simulink model "TosAeb"  (R2021a)
settings: Solver=FixedStepDiscrete  StartTime=0.0  StopTime=inf  FixedStep=0.01  RelTol=1e-3
undefined symbols (not defined in the file or in its folder): ACCModSts, AccSts1, AEB_ObjectMovingOrientation, AEB_ObjectMovingState, CN_BTCActive, CN_CurrentGearR, CN_CurrentGearR_DHT, CN_PTCActive, CN_VDCActive, CN_VSC_EspOff, CN_VSC_FCW_AEB_Error, CN_VSC_FCW_AEB_NoDispay, CN_VSC_FCW_AEB_PerfmDgdtn, CN_VSC_FCW_AEB_TempUnAvlbl, CN_VSC_VehStandstill, CNaccelAvoidMax, CNaccelAvoidMin, CNbrakePedalPress, CNcomfortStrLeftAccelMax, CNcomfortStrLeftAccelMin, CNcomfortStrRightAccelMax, CNcomfortStrRightAccelMin, CNDrvDoorOpen, CNDrvModeEco, CNDrvModeNorm, CNDrvModeOther, CNDrvModeSport, CNDrvSBRreminder, CNNull, CNobjInvalidID, CNPassDoorOpen, CNPassSBRreminder, CNPEBSAccelNegInf, CNPEBSAccelNull, CNPEBSTimeInf, CNPEBSTimeNull, CNSTOPMAX, CNSTOPNULL, CNsubjWidth, CNSwtOFF, CNSwtON, CNTTCMAX, CNTTCNULL, CNVehConfigAEBCycNone, CNVehConfigAEBNone, CNVehConfigAEBPedNone, CNVehConfigFCWNone, CNVehConfigJANone, CollisionSeverity, FALSE, FCWSnvty, JACycObjCalcInfoDefaultParams, JAPedObjCalcInfoDefaultParams, JAVehObjCalcInfoDefaultParams, latCycObjCalcInfoDefaultParams, latPedObjCalcInfoDefaultParams, longObjCalcInfoDefaultParams, ModSts, movingStatus, ObjectType ... (+1112)
libraries: simulink x3031, AEBSFunctionLib x50, Ta_Library x33, AEBSStateMachineLib x23, Mylibrary x12, simulink_extras x7, ObjSelect_lib x3, dspstat3 x2
(part 75 of 102)

== system TosAeb/AEB_Objsel/TA_Processor/AEB_ObjSelect/TargetInfoCheck/Data_precess/TA_EffectCalculate/Obj_FilterFlgCalculate/Long_ObjFilter/TTR_Calculate/CalculateTTRAndTTM_else/LongitudinalTTRComputer/DetermineLongitudinalClosestSide ==
  note: This is to avoid
      | floating-point equality.
  note: VLgtRel = VLgtObj - VLgtHost
  note: If host drives faster than the object, or if VRel == 0 and the object is located in front of host,
      | then host's front will meet the object first.
  PosnLgt : Inport
  VLgtRel : Inport  Port=2
  Compare To Zero1 : Subsystem  mask(Compare To Zero){relop=<, OutDataTypeStr=boolean, ZeroCross=on}
      mask prompts: relop "Simulink:masks:Operator_MP"; OutDataTypeStr "Simulink:masks:OutputDataType_MP"; ZeroCross "Simulink:masks:EnableZerocrossingDetection_MP"
      mask help: Simulink:masks:CompareToZero_MD
      mask init: same as TosAeb/AEB_Objsel/TA_Processor/AEB_ObjSelect/TargetInfoCheck/Data_precess/TA_EffectCalculate/Obj_FilterFlgCalculate/Long_ObjFilter/TTR_Calculate/CalculateTTRAndTTM_else/ModifyTTRAtSameSpeedAndAcceleration/Compare To Zero
      mask TypeOptions relop: == | ~= | < | <= | >= | >
      mask TypeOptions OutDataTypeStr: Simulink:masks:uint8 | Simulink:masks:boolean
      mask Callback ZeroCross: sl('zeroCrossEnable', gcbh);
      mask Display: disp([relop,' 0'])
  Compare To Zero5 : Subsystem  mask(Compare To Zero){relop=>, OutDataTypeStr=boolean, ZeroCross=on}
      mask prompts: relop "Simulink:masks:Operator_MP"; OutDataTypeStr "Simulink:masks:OutputDataType_MP"; ZeroCross "Simulink:masks:EnableZerocrossingDetection_MP"
      mask help: Simulink:masks:CompareToZero_MD
      mask init: same as TosAeb/AEB_Objsel/TA_Processor/AEB_ObjSelect/TargetInfoCheck/Data_precess/TA_EffectCalculate/Obj_FilterFlgCalculate/Long_ObjFilter/TTR_Calculate/CalculateTTRAndTTM_else/ModifyTTRAtSameSpeedAndAcceleration/Compare To Zero
      mask TypeOptions relop: == | ~= | < | <= | >= | >
      mask TypeOptions OutDataTypeStr: Simulink:masks:uint8 | Simulink:masks:boolean
      mask Callback ZeroCross: sl('zeroCrossEnable', gcbh);
      mask Display: disp([relop,' 0'])
  Logop_1 : Logic  AllPortsSameDT=off  OutDataTypeStr=boolean
  Logop_2 : Logic  Operator=OR  AllPortsSameDT=off  OutDataTypeStr=boolean
  Logop_3 : Logic  Operator=NOT  AllPortsSameDT=off  OutDataTypeStr=boolean
  SizeChecker : Subsystem  mask(Size checker){UseInput=off, Limit=1e-2, DataTypeIn=single, Operator=>}
      mask prompts: UseInput "Take the limit as an input"; DataTypeIn "Data type:"; Operator "Relational operator:"
      mask help: The block tests the absolute value of the supplied signal against a specified value using the selected operator. If the signal conforms to the test, true is returned; else, false is returned. The limit can either be set in the mask or as an external signal.
      mask init: same as TosAeb/AEB_Objsel/TA_Processor/AEB_ObjSelect/TargetInfoCheck/Data_precess/TA_EffectCalculate/Obj_FilterFlgCalculate/Long_ObjFilter/TTR_Calculate/CalculateTTRAndTTM_else/ModifyTTRAtSameSpeedAndAcceleration/SizeChecker
      settings: AttributesFormatString=DT=%<DataTypeIn>
      mask Help: The block tests the absolute value of the supplied signal against a specified value using the selected operator. If the signal conforms to the test, true is returned; else, false is returned. The limit can either be set in the mask or as an external signal.
      mask Callback UseInput:
      | % Author: Jakob Andersson (jande573)
      | 
      | % Enable and disable the box for the limit variable depending on the status of the checkbox
      | if strcmp(get_param(gcb,'UseInput'),'on')
      |     % Disable the box
      |     set_param(gcb,'MaskEnables',{'on','off','on','on'}); % One setting for each parameter in this mask; needs to be updated if the parameters are changed
      | else
      |     % Enable the box
      |     set_param(gcb,'MaskEnables',{'on','on','on','on'}); % One setting for each parameter in this mask; needs to be updated if the parameters are changed
      | end
      mask Callback Limit:
      | % Author: Jakob Andersson (jande573)
      | 
      | % Define the default value to apply if the entered value is disallowed
      | default='1e-6';
      | 
      | % Evaluate the entered value
      | val=eval(get_param(gcb,'Limit'));
      | 
      | % Test the entered value and revert to the default if necessary
      | if isnumeric(val)==false
      |     % Set the default if the entered value did not evaluate to a numeric
      |     set_param(gcb,'Limit',default);
      |     errordlg('The limit must be numeric. The default value will be used instead.');
      | elseif val<0
      |     % Set the default if the entered value is negative
      |     set_param(gcb,'Limit',default);
      |     errordlg('The limit must be positive since it is compared to an absolute value. The default value will be used instead.');
      | end
      mask TypeOptions Operator:
      | Relational
      | Operator/Operator
      mask Callback Operator:
      | % Author: Jakob Andersson (jande573)
      | 
      | % Define the default value to apply if the entered value is disallowed
      | EnabledOperators={'==','~=','<','<=','>=','>'};
      | default='>';
      | 
      | % Evaluate the selected operator and determine if it is ok
      | ok=any(strcmp(get_param(gcb,'Operator'),EnabledOperators));
      | 
      | % Revert the operator if it is disabled
      | if ~ok
      |     % Set the default if the entered value did not evaluate to a numeric
      |     set_param(gcb,'Operator',default);
      |     errordlg('The selected operator is disabled. The default operator will be used instead.');
      | end
      mask Display:
      | % Display the mask for this block
      | % Author: Jakob Andersson (jande573)
      | 
      | % Transform the operator to TeX
      | switch Operator
      |     case '=='
      |         op='=';
      |     case '~='
      |         op='\neq';
      |     case '<='
      |         op='\leq';
      |     case '>='
      |         op='\geq';
      |     otherwise
      |         op=Operator;
      | end
      | 
      | % Display the correct mask depending on the number of inputs
      | if strcmp(UseInput,'on')
      |     % Display the block logic and the set limit
      |     disp(horzcat('|u| ',op,' a'),'texmode','on');
      | 
      |     % Display the port title
      |     port_label('input',1,'u');
      |     port_label('input',2,'a');
      | else
      |     % Display the block logic and the set limit
      |     disp(horzcat('|u| ',op,' ',num2str(Limit)),'texmode','on');
      | 
      |     % Display the port title
      |     port_label('input',1,'u');
      | end
  HostFrontIsClosest : Outport
  wires:
  Compare To Zero1/1(y) -> Logop_2/1  "HostIsFastest"
  Logop_1/1 -> Logop_2/2
  Compare To Zero5/1(y) -> Logop_1/2  "ObjectInFrontOfHost"
  PosnLgt/1 -> Compare To Zero5/1(u)
  Logop_3/1 -> Logop_1/1  "SameSpeed"
  VLgtRel/1 -> Compare To Zero1/1(u), SizeChecker/1(Signal)
  SizeChecker/1(TestOk) -> Logop_3/1
  Logop_2/1 -> HostFrontIsClosest/1  "HostFrontIsClosest"

== system TosAeb/AEB_Objsel/TA_Processor/AEB_ObjSelect/TargetInfoCheck/Data_precess/TA_EffectCalculate/Obj_FilterFlgCalculate/Long_ObjFilter/TTR_Calculate/CalculateTTRAndTTM_else/LongitudinalTTRComputer/FindWhichMotionDataToUse ==
  note: If host's front is closest to the object now, then we expect that it will still be closest when the edges of host and object meet.
      | Or, if the edges have already met in the past, we assume that the front was meeting the object first.
      | Consequently, MotionDataFrontClosest and EdgeDistanceFrontClosest is the normal setup for the front closest case.
      |
      | If the relative velocity changes direction before host and object meet, no solution for TTI will be found with the normal setup.
      | Then the alternative setup must be used. For the front closest case, MotionDataRearClosest and EdgeDistanceRearClosest
      | is the alternative setup.
  HostFrontIsClosest : Inport
  MotionDataFrontClosest : Inport  Port=2
  MotionDataRearClosest : Inport  Port=3
  EdgeDistanceFrontClosest : Inport  Port=4
  EdgeDistanceRearClosest : Inport  Port=5
  Switch_{1..4} (4 alike) : Switch  Criteria=u2 ~= 0
  MotionDataNormalSetup : Outport
  MotionDataAlternativeSetup : Outport  Port=2
  EdgeDistanceNormalSetup : Outport  Port=3
  EdgeDistanceAlternativeSetup : Outport  Port=4
  wires:
  EdgeDistanceRearClosest/1 -> Switch_4/1, Switch_2/3
  EdgeDistanceFrontClosest/1 -> Switch_2/1, [EdgeFront]
  Switch_4/1 -> EdgeDistanceAlternativeSetup/1  "EdgeDistanceAlternativeSetup"
  [EdgeFront] -> Switch_4/3
  [MotionFront] -> Switch_3/3
  MotionDataRearClosest/1 -> Switch_3/1, Switch_1/3
  Switch_3/1 -> MotionDataAlternativeSetup/1  "MotionDataAlternativeSetup"
  [FrontClosest] -> Switch_4/2
  [FrontClosest] -> Switch_3/2
  Switch_2/1 -> EdgeDistanceNormalSetup/1  "EdgeDistanceNormalSetup"
  [FrontClosest] -> Switch_2/2
  Switch_1/1 -> MotionDataNormalSetup/1  "MotionDataNormalSetup"
  HostFrontIsClosest/1 -> Switch_1/2, [FrontClosest]
  MotionDataFrontClosest/1 -> Switch_1/1, [MotionFront]

== system TosAeb/AEB_Objsel/TA_Processor/AEB_ObjSelect/TargetInfoCheck/Data_precess/TA_EffectCalculate/Obj_FilterFlgCalculate/Long_ObjFilter/TTR_Calculate/CalculateTTRAndTTM_else/LongitudinalTTRComputer/SelectTTI ==
  MovingTTI : Inport
  StationaryHostTTI : Inport  Port=2
  StationaryObjectTTI : Inport  Port=3
  MovingSolutionExists : Inport  Port=4
  StationaryHostSolutionExists : Inport  Port=5
  StationaryObjectSolutionExists : Inport  Port=6
  StoppingData : Inport  Port=7
  BothHostAndObjectWillStop : Subsystem  TreatAsAtomicUnit=on
  Bus Selector : BusSelector  OutputSignals=HostStopTime,ObjectStopTime
  CheckIfVehicleStops : Subsystem
  CheckIfVehicleStops1 : Subsystem
  HostAndObjectAreMoving : Subsystem  TreatAsAtomicUnit=on
  HostOnlyWillStop : Subsystem  TreatAsAtomicUnit=on
  If : If  NumInputs=3  IfExpression=u1~=0  ElseIfExpressions=u2~=0,u3~=0
  Logop_3 : Logic  AllPortsSameDT=off  OutDataTypeStr=boolean
  Merge : Merge  Inputs=4
  Merge1 : Merge  Inputs=4
  ObjectOnlyWillStop : Subsystem  TreatAsAtomicUnit=on
  TimeToIntersection : Outport
  SolutionExists : Outport  Port=2
  wires:
  [StationaryHostSolutionExists_in] -> BothHostAndObjectWillStop/6(StationaryHostSolutionExists)
  [StationaryObjectSolutionExists_in] -> BothHostAndObjectWillStop/5(StationaryObjectSolutionExists)
  If/4 -> [HostAndObjectAreMoving]
  If/3 -> [ObjectOnlyWillStop]
  [ObjectStops] -> If/3
  If/2 -> [HostOnlyWillStop]
  If/1 -> [HostAndObjectWillStop]
  StationaryHostTTI/1 -> [StatHostTTI], CheckIfVehicleStops1/1(StationaryTTI)
  StationaryObjectTTI/1 -> CheckIfVehicleStops/1(StationaryTTI), [StatObjTTI]
  Logop_3/1 -> If/1  "BothHostAndObjectWillStop"
  BothHostAndObjectWillStop/1(TimeToIntersection) -> Merge/1
  Merge/1 -> TimeToIntersection/1  "TimeToIntersection"
  [ObjStopTime] -> BothHostAndObjectWillStop/3(ObjectStopTime)
  [StatObjTTI] -> ObjectOnlyWillStop/1(StationaryObjectTTI)
  [StatHostTTI] -> HostOnlyWillStop/1(StationaryHostTTI)
  [HostAndObjectWillStop] -> BothHostAndObjectWillStop/Action
  [StatHostTTI] -> BothHostAndObjectWillStop/2(StationaryHostTTI)
  [StatObjTTI] -> BothHostAndObjectWillStop/1(StationaryObjectTTI)
  [HostAndObjectAreMoving] -> HostAndObjectAreMoving/Action
  [HostOnlyWillStop] -> HostOnlyWillStop/Action
  [ObjectOnlyWillStop] -> ObjectOnlyWillStop/Action
  MovingTTI/1 -> [MovingTTI], HostAndObjectAreMoving/1(MovingTTI)
  Bus Selector/1 -> BothHostAndObjectWillStop/4(HostStopTime), CheckIfVehicleStops1/3(StopTime)  "<HostStopTime>"
  CheckIfVehicleStops1/1(VehicleStops) -> Logop_3/2, If/2  "HostStops"
  CheckIfVehicleStops/1(VehicleStops) -> [ObjectStops], Logop_3/1  "ObjectStops"
  StoppingData/1 -> Bus Selector/1
  Bus Selector/2 -> [ObjStopTime]  "<ObjectStopTime>"
  [ObjStopTime] -> CheckIfVehicleStops/3(StopTime)
  [MovingTTI] -> CheckIfVehicleStops/2(MovingTTI)
  [MovingTTI] -> CheckIfVehicleStops1/2(MovingTTI)
  MovingSolutionExists/1 -> [MovingSolutionExists_in]
  StationaryHostSolutionExists/1 -> [StationaryHostSolutionExists_in]
  StationaryObjectSolutionExists/1 -> [StationaryObjectSolutionExists_in]
  [StationaryObjectSolutionExists_in] -> ObjectOnlyWillStop/2(StationaryObjectSolutionExists)
  [StationaryHostSolutionExists_in] -> HostOnlyWillStop/2(StationaryHostSolutionExists)
  [MovingSolutionExists_in] -> HostAndObjectAreMoving/2(MovingSolutionExists)
  BothHostAndObjectWillStop/2(SolutionExists) -> [HostAndObjectSolution]
  HostOnlyWillStop/2(SolutionExists) -> [HostSolution]
  ObjectOnlyWillStop/2(SolutionExists) -> [ObjectSolution]
  HostAndObjectAreMoving/2(SolutionExists) -> Merge1/4
  HostOnlyWillStop/1(TimeToIntersection) -> Merge/2
  ObjectOnlyWillStop/1(TimeToIntersection) -> Merge/3
  HostAndObjectAreMoving/1(TimeToIntersection) -> Merge/4
  Merge1/1 -> SolutionExists/1  "SolutionExists"
  [HostAndObjectSolution] -> Merge1/1
  [HostSolution] -> Merge1/2
  [ObjectSolution] -> Merge1/3

== system TosAeb/AEB_Objsel/TA_Processor/AEB_ObjSelect/TargetInfoCheck/Data_precess/TA_EffectCalculate/Obj_FilterFlgCalculate/Long_ObjFilter/TTR_Calculate/CalculateTTRAndTTM_else/ModifyTTRAtSameSpeedAndAcceleration/Compare To Zero ==
  u : Inport
  Compare : RelationalOperator  Operator=<  OutDataTypeStr=boolean  RndMeth=Nearest  InputSameDT=on
  Constant : Constant  DisableCoverage=on  Value=0  OutDataTypeStr=Inherit: Inherit via back propagation  PreserveConstantTs=on
  y : Outport
  wires:
  Constant/1 -> Compare/2
  u/1 -> Compare/1
  Compare/1 -> y/1

== system TosAeb/AEB_Objsel/TA_Processor/AEB_ObjSelect/TargetInfoCheck/Data_precess/TA_EffectCalculate/Obj_FilterFlgCalculate/Long_ObjFilter/TTR_Calculate/CalculateTTRAndTTM_else/ModifyTTRAtSameSpeedAndAcceleration/Compare To Zero1 ==
  u : Inport
  Compare : RelationalOperator  Operator=<  OutDataTypeStr=boolean  RndMeth=Nearest  InputSameDT=on
  Constant : Constant  DisableCoverage=on  Value=0  OutDataTypeStr=Inherit: Inherit via back propagation  PreserveConstantTs=on
  y : Outport
  wires:
  Constant/1 -> Compare/2
  u/1 -> Compare/1
  Compare/1 -> y/1

== system TosAeb/AEB_Objsel/TA_Processor/AEB_ObjSelect/TargetInfoCheck/Data_precess/TA_EffectCalculate/Obj_FilterFlgCalculate/Long_ObjFilter/TTR_Calculate/CalculateTTRAndTTM_else/ModifyTTRAtSameSpeedAndAcceleration/SizeChecker ==
  Signal : Inport  OutDataTypeStr=DataTypeIn
      settings: AttributesFormatString=DT=%<OutDataTypeStr>
  Abs : Abs  SaturateOnIntegerOverflow=on
  LimitSource : Constant  Value=Limit  OutDataTypeStr=Inherit: Inherit via back propagation
      settings: AttributesFormatString=DT=%<OutDataTypeStr>
  Relational Operator : RelationalOperator  Operator=<  OutDataTypeStr=boolean  RndMeth=Nearest  InputSameDT=on
      settings: AttributesFormatString=InputSameDT=%<InputSameDT>
  TestOk : Outport  OutDataTypeStr=boolean
      settings: AttributesFormatString=DT=%<OutDataTypeStr>
  wires:
  Relational Operator/1 -> TestOk/1  "TestOk"
  Signal/1 -> Abs/1  "Signal"
  LimitSource/1 -> Relational Operator/2  "LimitSource"
  Abs/1 -> Relational Operator/1  "AbsoluteSignal"

== system TosAeb/AEB_Objsel/TA_Processor/AEB_ObjSelect/TargetInfoCheck/Data_precess/TA_EffectCalculate/Obj_FilterFlgCalculate/Long_ObjFilter/TTR_Calculate/CalculateTTRAndTTM_else/ModifyTTRAtSameSpeedAndAcceleration/SizeChecker1 ==
  Signal : Inport  OutDataTypeStr=DataTypeIn
      settings: AttributesFormatString=DT=%<OutDataTypeStr>
  Abs : Abs  SaturateOnIntegerOverflow=on
  LimitSource : Constant  Value=Limit  OutDataTypeStr=Inherit: Inherit via back propagation
      settings: AttributesFormatString=DT=%<OutDataTypeStr>
  Relational Operator : RelationalOperator  Operator=<  OutDataTypeStr=boolean  RndMeth=Nearest  InputSameDT=on
      settings: AttributesFormatString=InputSameDT=%<InputSameDT>
  TestOk : Outport  OutDataTypeStr=boolean
      settings: AttributesFormatString=DT=%<OutDataTypeStr>
  wires:
  Relational Operator/1 -> TestOk/1  "TestOk"
  Signal/1 -> Abs/1  "Signal"
  LimitSource/1 -> Relational Operator/2  "LimitSource"
  Abs/1 -> Relational Operator/1  "AbsoluteSignal"

== system TosAeb/AEB_Objsel/TA_Processor/AEB_ObjSelect/TargetInfoCheck/Data_precess/TA_EffectCalculate/Obj_FilterFlgCalculate/Long_ObjFilter/TTR_Calculate/CalculateTTRAndTTM_else/Obj_AbsInfo/InforForTTICalculation ==
  note: The MotionDataFrontClosest bus is used when host's front will meet the object first,
      | i.e. when host has the highest speed.
      | This set of data is also used when VRel == 0 and the object is located in front of host.
  note: The MotionDataRearClosest bus is used when host's rear will meet the object first,
      | i.e. when the object has the highest speed.
      | This set of data is also used when VRel == 0 and the object is located behind host.
  VRel : Inport
  VLgtObj : Inport  Port=2
  VLgtHost : Inport  Port=3
  ARel : Inport  Port=4
  ALgtObj : Inport  Port=5
  ALgtHost : Inport  Port=6
  Bus Creator : BusCreator  Inputs=6
  Bus Creator1 : BusCreator  Inputs=6
  Unary Minus{,1..5} (6 alike) : UnaryMinus
  MotionDataFrontClosest : Outport
  MotionDataRearClosest : Outport  Port=2
  wires:
  [ALgtHost] -> Bus Creator1/6  "ARelStationaryObject"
  [VRel] -> Unary Minus2/1
  Bus Creator/1 -> MotionDataFrontClosest/1  "MotionDataFrontClosest"
  Unary Minus1/1 -> Bus Creator/6  "ARelStationaryObject"
  ALgtObj/1 -> Bus Creator/5, [ALgtObj]  "ARelStationaryHost"
  [ALgtObj] -> Unary Minus5/1
  VLgtObj/1 -> Bus Creator/2, [VLgtObj]  "VRelStationaryHost"
  Unary Minus3/1 -> Bus Creator1/2  "VRelStationaryHost"
  ALgtHost/1 -> [ALgtHost], Unary Minus1/1
  [VLgtObj] -> Unary Minus3/1
  Unary Minus5/1 -> Bus Creator1/5  "ARelStationaryHost"
  Unary Minus2/1 -> Bus Creator1/1  "VRel"
  Unary Minus4/1 -> Bus Creator1/4  "ARel"
  [VLgtHost] -> Bus Creator1/3  "VRelStationaryObject"
  ARel/1 -> Unary Minus4/1, Bus Creator/4  "ARel"
  Unary Minus/1 -> Bus Creator/3  "VRelStationaryObject"
  VRel/1 -> [VRel], Bus Creator/1  "VRel"
  Bus Creator1/1 -> MotionDataRearClosest/1  "MotionDataRearClosest"
  VLgtHost/1 -> [VLgtHost], Unary Minus/1

== system TosAeb/AEB_Objsel/TA_Processor/AEB_ObjSelect/TargetInfoCheck/Data_precess/TA_EffectCalculate/Obj_FilterFlgCalculate/Long_ObjFilter/TTR_Calculate/CalculateTTRAndTTM_else/Obj_AbsInfo/LongitudinalEdgeToEdgeDistances ==
  note: This subsystem calculates the edge to edge distances for the following two cases:
      | 	* Host's front will meet the object first. This happens when host has the highest speed.
      | 	* Host's rear end will meet the object first. This happens when the object has the highest speed.
  TA_ObjInfo : Inport
  Add2 : Sum
  Bus Selector : BusSelector  OutputSignals=TA_ObsDx
  Constant : Constant  Value=P_TA_PrmForVehLongth
  Rename : Subsystem  mask(Rename){}
      mask help: Block intended for fooling the model advisor, which want the signal names to match the port names and which only allows signal renaming in combination with transformative operations. The block has no effect on the signal and is intended to replace, e.g., gain blocks with one as its multiplication factor.
      mask Help: Block intended for fooling the model advisor, which want the signal names to match the port names and which only allows signal renaming in combination with transformative operations. The block has no effect on the signal and is intended to replace, e.g., gain blocks with one as its multiplication factor.
      mask Display:
      | % Author: Jakob Andersson (jande573)
      | 
      | % Display the block title
      | disp('Rename');
  Rename1 : Subsystem  mask(Rename){}
      mask help: Block intended for fooling the model advisor, which want the signal names to match the port names and which only allows signal renaming in combination with transformative operations. The block has no effect on the signal and is intended to replace, e.g., gain blocks with one as its multiplication factor.
      mask Help: Block intended for fooling the model advisor, which want the signal names to match the port names and which only allows signal renaming in combination with transformative operations. The block has no effect on the signal and is intended to replace, e.g., gain blocks with one as its multiplication factor.
      mask Display:
      | % Author: Jakob Andersson (jande573)
      | 
      | % Display the block title
      | disp('Rename');
  Unary Minus6 : UnaryMinus
  CloseEdgeDistanceFrontClosest : Outport
  CloseEdgeDistanceRearClosest : Outport  Port=2
  FarEdgeDistanceFrontClosest : Outport  Port=3
  wires:
  Rename1/1(Name_out) -> FarEdgeDistanceFrontClosest/1  "FarEdgeDistanceFrontClosest"
  Unary Minus6/1 -> CloseEdgeDistanceRearClosest/1  "CloseEdgeDistanceRearClosest"
  Rename/1(Name_out) -> CloseEdgeDistanceFrontClosest/1  "CloseEdgeDistanceFrontClosest"
  Add2/1 -> Rename1/1(Name_in), Unary Minus6/1  "DistanceFromHostRear"
  Constant/1 -> Add2/2
  TA_ObjInfo/1 -> Bus Selector/1
  Bus Selector/1 -> Add2/1, Rename/1(Name_in)  "<TA_ObsDx>"

== system TosAeb/AEB_Objsel/TA_Processor/AEB_ObjSelect/TargetInfoCheck/Data_precess/TA_EffectCalculate/Obj_FilterFlgCalculate/Long_ObjFilter/TTR_Calculate/CalculateTTRAndTTM_else/Obj_AbsInfo/LongitudinalMotionData ==
  TA_ObjInfo : Inport
  VehSelfData : Inport  Port=2
  Bus Selector : BusSelector  OutputSignals=VehSelf.ABS_VehicleSpeed,VehSelf.AX1
  Bus Selector2 : BusSelector  OutputSignals=TA_ObsVx,TA_ObsVxAbs,TA_ObsAx,TA_ObsAxAbs
  Subsystem : Subsystem  Commented=on
  Obj_RefVx : Outport
  Obj_AbsVx : Outport  Port=2
  Subj_Vx : Outport  Port=3
  Obj_RefAcclx : Outport  Port=4
  Obj_AbsAcclx : Outport  Port=5
  Subj_Ax : Outport  Port=6
  wires:
  TA_ObjInfo/1 -> Bus Selector2/1
  Bus Selector2/1 -> Obj_RefVx/1  "<TA_ObsVx>"
  Bus Selector2/2 -> Obj_AbsVx/1  "<TA_ObsVxAbs>"
  Bus Selector2/3 -> Obj_RefAcclx/1  "<TA_ObsAx>"
  Bus Selector2/4 -> Obj_AbsAcclx/1  "<TA_ObsAxAbs>"
  VehSelfData/1 -> Bus Selector/1
  Bus Selector/2 -> Subj_Ax/1  "<AX1>"
  Bus Selector/1 -> Subj_Vx/1  "<ABS_VehicleSpeed>"

== system TosAeb/AEB_Objsel/TA_Processor/AEB_ObjSelect/TargetInfoCheck/Data_precess/TA_EffectCalculate/Obj_FilterFlgCalculate/Long_ObjFilter/TTR_Calculate/CalculateTTRAndTTM_else/Obj_AbsInfo/StoppingDataForTTICalculation ==
  note: 计算自车刹停时间、刹停距离
      | 计算目标刹停时间、刹停距离
  VLgtObj : Inport
  ALgtObj : Inport  Port=2
  VLgtHost : Inport  Port=3
  ALgtHost : Inport  Port=4
  TA_ObjInfo : Inport  Port=5
  Bus Creator : BusCreator  Inputs=4
  Bus Selector : BusSelector  OutputSignals=TA_ObsState
  Compare To Constant : simulink/Logic and Bit Operations/Compare To Constant  relop===  const=2  OutDataTypeStr=boolean
  Compare To Constant1 : simulink/Logic and Bit Operations/Compare To Constant  relop===  const=3  OutDataTypeStr=boolean
  Constant1 : Constant  Value=single(0)
  Constant2 : Constant  Value=single(0.01)
  Constant3 : Constant  Value=single(0)
  Constant4 : Constant  Value=single(0)
  Gain_1 : Gain  Gain=-1  ParamDataTypeStr=single  OutDataTypeStr=single
  Gain_2 : Gain  Gain=-1  ParamDataTypeStr=single  OutDataTypeStr=single
  LimitedSafeDivide : Subsystem
  LimitedSafeDivide1 : Subsystem
  LinearObjectPositionPredictor : Subsystem
  LinearObjectPositionPredictor1 : Subsystem
  Logical Operator : Logic  Operator=OR  AllPortsSameDT=off  OutDataTypeStr=boolean
  Switch : Switch  Criteria=u2 ~= 0
  Switch1 : Switch  Criteria=u2 ~= 0
  StoppingData : Outport
  wires:
  Gain_2/1 -> LimitedSafeDivide1/2(Denominator)  "Deceleration"
  Gain_1/1 -> LimitedSafeDivide/2(Denominator)  "Deceleration"
  VLgtObj/1 -> LinearObjectPositionPredictor1/2(V), LimitedSafeDivide/1(Numerator)
  LimitedSafeDivide/1(LimitedQuotient) -> LinearObjectPositionPredictor1/4(Ti), Switch/3  "ObjectStopTime"
  ALgtObj/1 -> LinearObjectPositionPredictor1/3(A), Gain_1/1
  VLgtHost/1 -> LimitedSafeDivide1/1(Numerator), LinearObjectPositionPredictor/2(V)
  LimitedSafeDivide1/1(LimitedQuotient) -> [HostStopTime], Bus Creator/3  "HostStopTime"
  ALgtHost/1 -> Gain_2/1, LinearObjectPositionPredictor/3(A)
  LinearObjectPositionPredictor/1(PosnPred) -> Bus Creator/4  "HostDeltaPos"
  Constant4/1 -> LinearObjectPositionPredictor/1(Posn)
  LinearObjectPositionPredictor1/1(PosnPred) -> Switch1/3  "ObjectDeltaPos"
  Constant1/1 -> LinearObjectPositionPredictor1/1(Posn)
  [HostStopTime] -> LinearObjectPositionPredictor/4(Ti)
  Bus Creator/1 -> StoppingData/1  "StoppingData"
  [Stationary] -> Switch/2
  Constant2/1 -> Switch/1
  [Stationary] -> Switch1/2
  Constant3/1 -> Switch1/1
  Switch1/1 -> Bus Creator/1  "ObjectDeltaPos"
  Switch/1 -> Bus Creator/2  "ObjectStopTime"
  TA_ObjInfo/1 -> Bus Selector/1
  Compare To Constant/1 -> Logical Operator/1
  Logical Operator/1 -> [Stationary]
  Compare To Constant1/1 -> Logical Operator/2
  Bus Selector/1 -> Compare To Constant1/1, Compare To Constant/1  "<TA_ObsState>"

== system TosAeb/AEB_Objsel/TA_Processor/AEB_ObjSelect/TargetInfoCheck/Data_precess/TA_EffectCalculate/Obj_FilterFlgCalculate/Long_ObjFilter/TTR_Calculate/CalculateTTRAndTTM_else/SetCorrectSign/Compare To Zero1 ==
  u : Inport
  Compare : RelationalOperator  Operator=<  OutDataTypeStr=boolean  RndMeth=Nearest  InputSameDT=on
  Constant : Constant  DisableCoverage=on  Value=0  OutDataTypeStr=Inherit: Inherit via back propagation  PreserveConstantTs=on
  y : Outport
  wires:
  Constant/1 -> Compare/2
  u/1 -> Compare/1
  Compare/1 -> y/1

== system TosAeb/AEB_Objsel/TA_Processor/AEB_ObjSelect/TargetInfoCheck/Data_precess/TA_EffectCalculate/Obj_FilterFlgCalculate/Long_ObjFilter/TTR_Calculate/CalculateTTRAndTTM_else/SetCorrectSign/Compare To Zero2 ==
  u : Inport
  Compare : RelationalOperator  Operator=<  OutDataTypeStr=boolean  RndMeth=Nearest  InputSameDT=on
  Constant : Constant  DisableCoverage=on  Value=0  OutDataTypeStr=Inherit: Inherit via back propagation  PreserveConstantTs=on
  y : Outport
  wires:
  Constant/1 -> Compare/2
  u/1 -> Compare/1
  Compare/1 -> y/1

== system TosAeb/AEB_Objsel/TA_Processor/AEB_ObjSelect/TargetInfoCheck/Data_precess/TA_EffectCalculate/ValideateObj/ObjPos_Calculate/ObjPos_Calculateelse/InPath_Cal/DetermineInPath/Compare To Zero ==
  u : Inport
  Compare : RelationalOperator  Operator=<  OutDataTypeStr=boolean  RndMeth=Nearest  InputSameDT=on
  Constant : Constant  DisableCoverage=on  Value=0  OutDataTypeStr=Inherit: Inherit via back propagation  PreserveConstantTs=on
  y : Outport
  wires:
  Constant/1 -> Compare/2
  u/1 -> Compare/1
  Compare/1 -> y/1

== system TosAeb/AEB_Objsel/TA_Processor/AEB_ObjSelect/TargetInfoCheck/Data_precess/TA_EffectCalculate/ValideateObj/ObjPos_Calculate/ObjPos_Calculateelse/InPath_Cal/DetermineInPath/LimitedSafeDivide ==
  Numerator : Inport
  Denominator : Inport  Port=2
  Constant1 : Constant  Value=-1000  OutDataTypeStr=single
  Constant5 : Constant  Value=1000  OutDataTypeStr=single
  SafeDivide : Subsystem  TreatAsAtomicUnit=on  mask(Safe divide){DataType=single, DataTypeMax=0, DataTypeMin=0}
      mask prompts: DataType "Data type:"
      mask help:
      | This block offers division protected from division by zero.
      |
      | When division by zero is detected, a very large number will be returned with the same sign as the numerator.
      |
      | In the case zero divided by zero, the output has been defined as zero.
      |
      | Both floating-point numbers and integers are supported.
      mask init: same as TosAeb/AEB_Objsel/TA_Processor/PreProcessor/VehiclePreProcessor/CalculateCurvature/SafeDivide
      settings: RTWSystemCode=Inline
      mask Help:
      | This block offers division protected from division by zero.
      | 
      | When division by zero is detected, a very large number will be returned with the same sign as the numerator.
      | 
      | In the case zero divided by zero, the output has been defined as zero.
      | 
      | Both floating-point numbers and integers are supported.
      mask Display:
      | % Author: Jakob Andersson (jande573)
      | 
      | % Display the allowed data type
      | disp(DataType);
      | 
      | % Display the port symbols
      | port_label('input',1,'\bullet','texmode','on');
      | port_label('input',2,'\div','texmode','on');
  Saturation Dynamic : Subsystem  mask(Saturation Dynamic){OutMin=[], OutMax=[], OutDataTypeStr=Inherit: Same as second input, OutputDataTypeScalingMode=Same as second input, OutDataType=fixdt(1, 16), OutScaling=2^-10, LockScale=off, RndMeth=Floor, DoSatur=off}
      mask prompts: OutMin "Simulink:masks:OutputMinimum_MP"; OutMax "Simulink:masks:OutputMaximum_MP"; OutDataTypeStr "Simulink:masks:OutputDataType_MP"; OutputDataTypeScalingMode "Simulink:masks:OutputDataTypeMode_MP"; OutDataType "Simulink:masks:OutputDataTypeegFixdt116Fixdtsingle_MP"; OutScaling "Simulink:masks:OutputScalingSlopeEg29OrSlopeBias_MP"; LockScale "Simulink:masks:LockOutputDataTypeAgainstFxpTools_MP"; RndMeth "Simulink:masks:IntegerRoundingMode_MP"; DoSatur "Simulink:masks:SaturateOnIntegerOverflow_MP"
      mask help: Simulink:masks:SaturationDynamic_MD
      mask init:
      | blkH = gcbh;
      | blkName = getfullname(blkH);
      | 
      | switch_blks = {'Switch','Switch2'}; 
      | for i = 1:length(switch_blks)
      |   set_param([blkName,'/',switch_blks{i}], ...
      |       'RndMeth', RndMeth, ...
      | 	  'DoSatur', DoSatur);
      | end
      | 
      | propBlk = [blkName, '/Data Type Propagation'];
      | 
      | if strcmp(OutputDataTypeScalingMode, 'Same as second input')
      |     set_param(propBlk, 'PropDataTypeMode', 'Inherit via propagation rule', ...
      |                        'PropScalingMode',  'Inherit via propagation rule');
      | else
      |     scaling = OutScaling;
      |     scalingMode = 'Specify via dialog';
      |     switch OutputDataTypeScalingMode
      |       case 'double'
      |         dataType = 'fixdt(''double'')';
      |       case 'single'
      |         dataType = 'fixdt(''single'')';
      |       case 'int8'
      |         dataType = 'fixdt(1,8,0)';
      |       case 'uint8'
      |         dataType = 'fixdt(0,8,0)';
      |       case 'int16'
      |         dataType = 'fixdt(1,16,0)';
      |       case 'uint16'
      |         dataType = 'fixdt(0,16,0)';
      |       case 'int32'
      |         dataType = 'fixdt(1,32,0)';
      |       case 'uint32'
      |         dataType = 'fixdt(0,32,0)';
      |       case 'Specify via dialog'
      |         dataType = OutDataType;
      |         if ~isempty(OutDataTypeStr) && isa(OutDataTypeStr,'Simulink.NumericType') 
      |           if strcmp(OutDataTypeStr.DataTypeMode, 'Fixed-point: unspecified scaling') 
      |             scalingMode = 'Obtain via best precision';
      |             range = [OutMin OutMax];
      |             range = range(~isinf(range));
      |             set_param(propBlk, 'ValuesUsedBestPrec', mat2str(range));
      |           end
      |         end
      |     end
      |     
      |     set_param(propBlk, 'PropDataTypeMode', 'Specify via dialog', ...
      |                        'PropScalingMode',  scalingMode, ...
      |                        'PropDataType',     dataType, ...
      |                        'PropScaling',      scaling);
      | end
      mask Callback OutDataTypeStr: fixptdialog(gcbh)
      mask TypeOptions OutputDataTypeScalingMode: double | single | int8 | uint8 | int16 | uint16 | int32 | uint32 | Specify via dialog | Same as second input
      mask Callback OutputDataTypeScalingMode: fixptdialog(gcbh)
      mask Callback OutDataType: fixptdialog(gcbh)
      mask TypeOptions RndMeth: Simulink:masks:Ceiling | Simulink:masks:Convergent | Simulink:masks:Floor | Simulink:masks:Nearest | Simulink:masks:Round | Simulink:masks:Simplest | Simulink:masks:Zero
      mask Display: plot([0.15 0.85],[.5 .5],[.5 .5],[0.05 0.95],0.15+0.7*[0 .25 .75 1],0.05+0.9*[.25 .25 .75 .75]);
  LimitedQuotient : Outport
  wires:
  Constant1/1 -> Saturation Dynamic/3(lo)
  Constant5/1 -> Saturation Dynamic/1(up)
  Saturation Dynamic/1(y) -> LimitedQuotient/1
  SafeDivide/1(Quotient) -> Saturation Dynamic/2(u)
  Denominator/1 -> SafeDivide/2(Denominator)
  Numerator/1 -> SafeDivide/1(Numerator)

== system TosAeb/AEB_Objsel/TA_Processor/AEB_ObjSelect/TargetInfoCheck/Data_precess/TA_EffectCalculate/ValideateObj/ObjPos_Calculate/ObjPos_Calculateelse/InPath_Cal/DetermineInPath/PredictHostLateralPositionAtTTR ==
  PredictionTime : Inport  PortDimensions=1
  PahEstimn : Inport  Port=2
  CalculateHeading : Subsystem  Commented=on
  PositionAndDerivative : MATLAB Function  TreatAsAtomicUnit=on
  PositionAndDerivative1 : MATLAB Function  TreatAsAtomicUnit=on
  SegmentComponents : Subsystem
  SelectSegment : Subsystem
  PredHostPosnLgt : Outport
  PredHostPosnLat : Outport  Port=2
  PredHostHeading : Outport  Port=3
  wires:
  [tau] -> PositionAndDerivative/5(tau)
  PositionAndDerivative1/1(y) -> PredHostPosnLat/1  "PredHostPosnLat"
  PositionAndDerivative/1(x) -> PredHostPosnLgt/1  "PredHostPosnLgt"
  SegmentComponents/9(tau) -> [tau], PositionAndDerivative1/5(tau)
  SegmentComponents/8(P3y) -> PositionAndDerivative1/4(P3)
  SegmentComponents/7(P2y) -> PositionAndDerivative1/3(P2)
  SegmentComponents/6(P1y) -> PositionAndDerivative1/2(P1)
  SegmentComponents/5(P0y) -> PositionAndDerivative1/1(P0)
  SegmentComponents/4(P3x) -> PositionAndDerivative/4(P3)
  SegmentComponents/3(P2x) -> PositionAndDerivative/3(P2)
  SegmentComponents/2(P1x) -> PositionAndDerivative/2(P1)
  SegmentComponents/1(P0x) -> PositionAndDerivative/1(P0)
  PahEstimn/1 -> SelectSegment/1(PahEstimn), SegmentComponents/1(Segment), CalculateHeading/1(PathEstimn)  "PahEstimn"
  PredictionTime/1 -> SegmentComponents/2(PredictionTime), SelectSegment/2(Time)  "PredictionTime"
  PositionAndDerivative/2(dx) -> [dx]
  PositionAndDerivative1/2(dy) -> [dy]
  CalculateHeading/1(PredHostHeading) -> PredHostHeading/1
  [dx] -> CalculateHeading/3(dx)
  [dy] -> CalculateHeading/4(dy)
  SelectSegment/1(SegmentIndex) -> SegmentComponents/3(SegmentIndex)

== system TosAeb/AEB_Objsel/TA_Processor/AEB_ObjSelect/TargetInfoCheck/Data_precess/TA_EffectCalculate/ValideateObj/ObjPos_Calculate/ObjPos_Calculateelse/InPath_Cal/DetermineInPath/PredictObjectLateralPositionAtTTR ==
  note: Constant acceleration prediction:
      | pos_pred = pos + vel*time + acc*time^2/2
      | vel_pred = vel + acc*time
      | acc_pred = acc
  Posn : Inport  OutDataTypeStr=single
      settings: AttributesFormatString=DT=%<OutDataTypeStr>
  V : Inport  Port=2  OutDataTypeStr=single
      settings: AttributesFormatString=DT=%<OutDataTypeStr>
  A : Inport  Port=3  OutDataTypeStr=single
      settings: AttributesFormatString=DT=%<OutDataTypeStr>
  Ti : Inport  Port=4  OutDataTypeStr=single
      settings: AttributesFormatString=DT=%<OutDataTypeStr>
  Constant : Constant  Value=single(0.5)  OutDataTypeStr=single
      settings: AttributesFormatString=DT=%<OutDataTypeStr>
  Product1 : Product  RndMeth=Zero
  Product4 : Product  Inputs=**  RndMeth=Zero
  Product5 : Product  Inputs=***  RndMeth=Zero
  Product6 : Product  Inputs=**  RndMeth=Zero
  Rename : Subsystem  mask(Rename){}
      mask help: Block intended for fooling the model advisor, which want the signal names to match the port names and which only allows signal renaming in combination with transformative operations. The block has no effect on the signal and is intended to replace, e.g., gain blocks with one as its multiplication factor.
      mask Help: Block intended for fooling the model advisor, which want the signal names to match the port names and which only allows signal renaming in combination with transformative operations. The block has no effect on the signal and is intended to replace, e.g., gain blocks with one as its multiplication factor.
      mask Display:
      | % Author: Jakob Andersson (jande573)
      | 
      | % Display the block title
      | disp('Rename');
  Sum3 : Sum
  Sum6 : Sum  Inputs=+++
  PosnPred : Outport  OutDataTypeStr=single
      settings: AttributesFormatString=DT=%<OutDataTypeStr>
  VPred : Outport  Port=2  OutDataTypeStr=single
      settings: AttributesFormatString=DT=%<OutDataTypeStr>
  APred : Outport  Port=3  OutDataTypeStr=single
      settings: AttributesFormatString=DT=%<OutDataTypeStr>
  wires:
  Rename/1(Name_out) -> APred/1  "APred"
  Product5/1 -> Sum6/3  "PositionChangeFromAcceleration"
  Constant/1 -> Product5/3  "Denominator"
  Posn/1 -> Sum6/1  "Posn"
  V/1 -> Product4/1, Sum3/1  "V"
  Ti/1 -> Product1/2, Product1/1, Product4/2, Product6/1  "Ti"
  A/1 -> Product6/2, Rename/1(Name_in), Product5/1  "A"
  Product4/1 -> Sum6/2  "PositionChangeFromVelocity"
  Product1/1 -> Product5/2  "TimeSquared"
  Sum6/1 -> PosnPred/1  "PosnPred"
  Product6/1 -> Sum3/2  "VelocityChangeFromAcceleration"
  Sum3/1 -> VPred/1  "VPred"

== system TosAeb/AEB_Objsel/TA_Processor/AEB_ObjSelect/TargetInfoCheck/Data_precess/TA_EffectCalculate/ValideateObj/ValidObj_PreProcess/If Action Subsystem1/CheckPosition/Subsystem/dxCalc_if ==
  ObsDx : Inport
  Action Port : ActionPort  ActionPortLabel=if(u1 > 0)
  Obsdx : Outport
  wires:
  ObsDx/1 -> Obsdx/1  "ObjPreSelectCond"

== system TosAeb/AEB_Objsel/TA_Processor/AEB_ObjSelect/TargetInfoCheck/Data_precess/TA_EffectCalculate/ValideateObj/ValidObj_PreProcess/If Action Subsystem1/CheckPosition/Subsystem/dxcalc ==
  Action Port : ActionPort  ActionPortLabel=else
  Constant : Constant  Value=0  OutDataTypeStr=single
  Obsdx : Outport
  wires:
  Constant/1 -> Obsdx/1  "ObjPreSelectCond"

== system TosAeb/AEB_Objsel/TA_Processor/PreProcessor/ObjectPrecessor/TAObjInfoCalc/For Each Subsystem/Info_Check1/BasicInfo_check/DxCorrect/dx_charge/Dx_Flag/dx_AebState ==
  Veh_AEBFunState : Inport
  Compare To Constant : simulink/Logic and Bit Operations/Compare To Constant  relop===  const=0  OutDataTypeStr=boolean
  AEB_State : Outport
  wires:
  Veh_AEBFunState/1 -> Compare To Constant/1
  Compare To Constant/1 -> AEB_State/1

== system TosAeb/AEB_Objsel/TA_Processor/PreProcessor/ObjectPrecessor/TAObjInfoCalc/For Each Subsystem/Info_Check1/BasicInfo_check/DxCorrect/dx_charge/Dx_Flag/dx_compare ==
  Fus_ObjDx_Single : Inport
  Fus_ObjVx_Single : Inport  Port=2
  DistancebyDx : Subsystem
  DistancebyVx : Subsystem
  distance_charge : Subsystem
  DisLong_flg : Outport
  wires:
  Fus_ObjDx_Single/1 -> DistancebyDx/1(Fus_ObjDx_Single)
  Fus_ObjVx_Single/1 -> DistancebyVx/1(Fus_ObjVx_Single)
  DistancebyDx/1(DisbyDx) -> distance_charge/1(DisbyDx)
  DistancebyVx/1(DisbyVx) -> distance_charge/2(DisbyVx)
  distance_charge/1(DisLong_flg) -> DisLong_flg/1

== system TosAeb/AEB_Objsel/TA_Processor/PreProcessor/ObjectPrecessor/TAObjInfoCalc/For Each Subsystem/Info_Check1/BasicInfo_check/DyCorrect/dy_charge/Subsystem2/Subsystem1 ==
  Veh_AEBFunState : Inport
  Compare To Constant : simulink/Logic and Bit Operations/Compare To Constant  relop===  const=0  OutDataTypeStr=boolean
  AEB_State : Outport
  wires:
  Compare To Constant/1 -> AEB_State/1
  Veh_AEBFunState/1 -> Compare To Constant/1

== system TosAeb/AEB_Objsel/TA_Processor/PreProcessor/ObjectPrecessor/TAObjInfoCalc/For Each Subsystem/Info_Check1/BasicInfo_check/DyCorrect/dy_charge/Subsystem2/charge ==
  Fus_ObjDy_Single : Inport
  Fus_ObjVy_Single : Inport  Port=2
  DistancebyDy : Subsystem
  DistancebyVy : Subsystem
  Subsystem : Subsystem
  DisLat_flg : Outport
  wires:
  Subsystem/1(DisLat_flg) -> DisLat_flg/1
  DistancebyVy/1(DisbyVy) -> Subsystem/2(DisbyVy)
  DistancebyDy/1(DisbyDy) -> Subsystem/1(DisbyDy)
  Fus_ObjVy_Single/1 -> DistancebyVy/1(Fus_ObjVy_Single)
  Fus_ObjDy_Single/1 -> DistancebyDy/1(Fus_ObjDyQua_Single)

== system TosAeb/AEB_Objsel/TA_Processor/PreProcessor/ObjectPrecessor/TAObjInfoCalc/For Each Subsystem/Info_Check1/BasicInfo_check/HeadAngle/If Action Subsystem1/HeadAngle_c/Subsystem2 ==
  Obj_vy : Inport
  Obj_Vx : Inport  Port=2
  Atan2 : Trigonometry  Operator=atan2
  Constant : Constant  Value=pi/2
  MapToAngularInterval : Ta_Library/MapToAngularInterval  SystemSampleTime=-1  Latency=0  AutoFrameSizeCalculation=off
  Obj_Head : Outport
  wires:
  Constant/1 -> MapToAngularInterval/2
  Obj_Vx/1 -> Atan2/2
  Obj_vy/1 -> Atan2/1
  Atan2/1 -> MapToAngularInterval/1
  MapToAngularInterval/1 -> Obj_Head/1

== system TosAeb/AEB_Objsel/TA_Processor/PreProcessor/ObjectPrecessor/TAObjInfoCalc/For Each Subsystem/Info_Check1/BasicInfo_check/QuaCorrect/quality_recrectionelse/Quality_else/Factor_Cal ==
  dy_flg : Inport
  dx_flg : Inport  Port=2
  vx_flg : Inport  Port=3
  vy_flg : Inport  Port=4
  Add : Sum  Inputs=++++
  Constant : Constant  Value=5  OutDataTypeStr=single
  Data Type Conversion{,1..3} (4 alike) : DataTypeConversion  OutDataTypeStr=single
  Product : Product
  factor : Outport
  wires:
  Data Type Conversion1/1 -> Add/2
  Data Type Conversion3/1 -> Add/4
  vy_flg/1 -> Data Type Conversion3/1
  dy_flg/1 -> Data Type Conversion/1
  Data Type Conversion/1 -> Add/1
  Add/1 -> Product/1
  dx_flg/1 -> Data Type Conversion1/1
  vx_flg/1 -> Data Type Conversion2/1
  Data Type Conversion2/1 -> Add/3
  Product/1 -> factor/1
  Constant/1 -> Product/2

== system TosAeb/AEB_Objsel/TA_Processor/PreProcessor/ObjectPrecessor/TAObjInfoCalc/For Each Subsystem/Info_Check1/BasicInfo_check/QuaCorrect/quality_recrectionelse/Quality_else/Quality_Out ==
  Fus_TargetQuality : Inport
  factor : Inport  Port=2
  Add : Sum  Inputs=+-
  Data Type Conversion : DataTypeConversion  OutDataTypeStr=single
  Data Type Conversion1 : DataTypeConversion  OutDataTypeStr=uint8
  Fus_ObjQuality : Outport
  wires:
  Data Type Conversion1/1 -> Fus_ObjQuality/1
  Add/1 -> Data Type Conversion1/1
  factor/1 -> Add/2
  Data Type Conversion/1 -> Add/1
  Fus_TargetQuality/1 -> Data Type Conversion/1

== system TosAeb/AEB_Objsel/TA_Processor/PreProcessor/ObjectPrecessor/TAObjInfoCalc/For Each Subsystem/Info_Check1/BasicInfo_check/VxCorrect/Subsystem3/Subsystem2/Subsystem ==
  Fus_ObjDx_Single : Inport
  Fus_ObjVx_Single : Inport  Port=2
  Subsystem : Subsystem
  VbyDx : Subsystem
  VbyVx : Subsystem
  DisLong_flg : Outport
  wires:
  Subsystem/1(DisLong_flg) -> DisLong_flg/1
  VbyVx/1(VbyVx) -> Subsystem/2(VbyVx)
  VbyDx/1(VbyDx) -> Subsystem/1(VbyDx)
  Fus_ObjVx_Single/1 -> VbyVx/1(Fus_ObjVx_Single)
  Fus_ObjDx_Single/1 -> VbyDx/1(Fus_ObjDx_Single)

== system TosAeb/AEB_Objsel/TA_Processor/PreProcessor/ObjectPrecessor/TAObjInfoCalc/For Each Subsystem/Info_Check1/BasicInfo_check/VxCorrect/Subsystem3/Subsystem2/Subsystem1 ==
  Veh_AEBFunState : Inport
  Compare To Constant : simulink/Logic and Bit Operations/Compare To Constant  relop===  const=0  OutDataTypeStr=boolean
  AEB_State : Outport
  wires:
  Compare To Constant/1 -> AEB_State/1
  Veh_AEBFunState/1 -> Compare To Constant/1

== system TosAeb/AEB_Objsel/TA_Processor/PreProcessor/ObjectPrecessor/TAObjInfoCalc/For Each Subsystem/Info_Check1/BasicInfo_check/VyCorrect/Subsystem3/Subsystem2/Subsystem ==
  Fus_ObjDy_Single : Inport
  Fus_ObjVy_Single : Inport  Port=2
  Subsystem : Subsystem
  VbyDy : Subsystem
  VbyVy : Subsystem
  DisLat_flg : Outport
  wires:
  Fus_ObjDy_Single/1 -> VbyDy/1(Fus_ObjDy_Single)
  Fus_ObjVy_Single/1 -> VbyVy/1(Fus_ObjVy_Single)
  VbyDy/1(VbyDy) -> Subsystem/1(VbyDy)
  VbyVy/1(VbyVy) -> Subsystem/2(VbyVy)
  Subsystem/1(DisLat_flg) -> DisLat_flg/1

== system TosAeb/AEB_Objsel/TA_Processor/PreProcessor/ObjectPrecessor/TAObjInfoCalc/For Each Subsystem/Info_Check1/BasicInfo_check/VyCorrect/Subsystem3/Subsystem2/Subsystem1 ==
  Veh_AEBFunState : Inport
  Compare To Constant : simulink/Logic and Bit Operations/Compare To Constant  relop===  const=1  OutDataTypeStr=boolean
  AEB_State : Outport
  wires:
  Veh_AEBFunState/1 -> Compare To Constant/1
  Compare To Constant/1 -> AEB_State/1

== system TosAeb/AEB_Objsel/TA_Processor/PreProcessor/ObjectPrecessor/TAObjInfoCalc/For Each Subsystem/Info_Check1/BasicInfo_check/VyCorrect/Subsystem3/Subsystem2/Subsystem2 ==
  Fus_TargetState : Inport
  Veh_YawRate : Inport  Port=2
  Abs : Abs
  Compare To Constant : simulink/Logic and Bit Operations/Compare To Constant  relop=<=  const=0.02  OutDataTypeStr=boolean
  Compare To Constant1 : simulink/Logic and Bit Operations/Compare To Constant  relop===  const=2  OutDataTypeStr=boolean
  Compare To Constant2 : simulink/Logic and Bit Operations/Compare To Constant  relop===  const=3  OutDataTypeStr=boolean
  Logical Operator : Logic  Operator=OR  AllPortsSameDT=off  OutDataTypeStr=boolean
  Logical Operator1 : Logic  AllPortsSameDT=off  OutDataTypeStr=boolean
  State_flg : Outport
  wires:
  Logical Operator1/1 -> State_flg/1
  Logical Operator/1 -> Logical Operator1/1
  Compare To Constant2/1 -> Logical Operator/2
  Compare To Constant1/1 -> Logical Operator/1
  Fus_TargetState/1 -> Compare To Constant1/1, Compare To Constant2/1
  Veh_YawRate/1 -> Abs/1
  Abs/1 -> Compare To Constant/1
  Compare To Constant/1 -> Logical Operator1/2

== system TosAeb/AEB_Objsel/TA_Processor/PreProcessor/ObjectPrecessor/TAObjInfoCalc/For Each Subsystem/Info_Check1/Basic_Info_check/DxCorrect/dx_charge/Dx_Flag/dx_AebState ==
  Veh_AEBFunState : Inport
  Compare To Constant : simulink/Logic and Bit Operations/Compare To Constant  relop===  const=0  OutDataTypeStr=boolean
  AEB_State : Outport
  wires:
  Veh_AEBFunState/1 -> Compare To Constant/1
  Compare To Constant/1 -> AEB_State/1

== system TosAeb/AEB_Objsel/TA_Processor/PreProcessor/ObjectPrecessor/TAObjInfoCalc/For Each Subsystem/Info_Check1/Basic_Info_check/DxCorrect/dx_charge/Dx_Flag/dx_compare ==
  Fus_ObjDx_Single : Inport
  Fus_ObjVx_Single : Inport  Port=2
  DistancebyDx : Subsystem
  DistancebyVx : Subsystem
  distance_charge : Subsystem
  DisLong_flg : Outport
  wires:
  Fus_ObjDx_Single/1 -> DistancebyDx/1(Fus_ObjDx_Single)
  DistancebyDx/1(DisbyDx) -> distance_charge/1(DisbyDx)
  DistancebyVx/1(DisbyVx) -> distance_charge/2(DisbyVx)
  distance_charge/1(DisLong_flg) -> DisLong_flg/1
  Fus_ObjVx_Single/1 -> DistancebyVx/1(Fus_ObjVx_Single)

== system TosAeb/AEB_Objsel/TA_Processor/PreProcessor/ObjectPrecessor/TAObjInfoCalc/For Each Subsystem/Info_Check1/Basic_Info_check/DxCorrect1/dx_charge/Dx_Flag/dx_AebState ==
  Veh_AEBFunState : Inport
  Compare To Constant : simulink/Logic and Bit Operations/Compare To Constant  relop===  const=0  OutDataTypeStr=boolean
  AEB_State : Outport
  wires:
  Compare To Constant/1 -> AEB_State/1
  Veh_AEBFunState/1 -> Compare To Constant/1

== system TosAeb/AEB_Objsel/TA_Processor/PreProcessor/ObjectPrecessor/TAObjInfoCalc/For Each Subsystem/Info_Check1/Basic_Info_check/DxCorrect1/dx_charge/Dx_Flag/dx_compare ==
  Fus_ObjDx_Single : Inport
  Fus_ObjVx_Single : Inport  Port=2
  DistancebyDx : Subsystem
  DistancebyVx : Subsystem
  distance_charge : Subsystem
  DisLong_flg : Outport
  wires:
  Fus_ObjVx_Single/1 -> DistancebyVx/1(Fus_ObjVx_Single)
  distance_charge/1(DisLong_flg) -> DisLong_flg/1
  DistancebyVx/1(DisbyVx) -> distance_charge/2(DisbyVx)
  DistancebyDx/1(DisbyDx) -> distance_charge/1(DisbyDx)
  Fus_ObjDx_Single/1 -> DistancebyDx/1(Fus_ObjDx_Single)

== system TosAeb/AEB_Objsel/TA_Processor/PreProcessor/ObjectPrecessor/TAObjInfoCalc/For Each Subsystem/Info_Check1/Basic_Info_check/DyCorrect/dy_charge/Subsystem2/Subsystem1 ==
  Veh_AEBFunState : Inport
  Compare To Constant : simulink/Logic and Bit Operations/Compare To Constant  relop===  const=0  OutDataTypeStr=boolean
  AEB_State : Outport
  wires:
  Compare To Constant/1 -> AEB_State/1
  Veh_AEBFunState/1 -> Compare To Constant/1

== system TosAeb/AEB_Objsel/TA_Processor/PreProcessor/ObjectPrecessor/TAObjInfoCalc/For Each Subsystem/Info_Check1/Basic_Info_check/DyCorrect/dy_charge/Subsystem2/charge ==
  Fus_ObjDy_Single : Inport
  Fus_ObjVy_Single : Inport  Port=2
  DistancebyDy : Subsystem
  DistancebyVy : Subsystem
  Subsystem : Subsystem
  DisLat_flg : Outport
  wires:
  Subsystem/1(DisLat_flg) -> DisLat_flg/1
  DistancebyVy/1(DisbyVy) -> Subsystem/2(DisbyVy)
  DistancebyDy/1(DisbyDy) -> Subsystem/1(DisbyDy)
  Fus_ObjVy_Single/1 -> DistancebyVy/1(Fus_ObjVy_Single)
  Fus_ObjDy_Single/1 -> DistancebyDy/1(Fus_ObjDyQua_Single)
